simulink model "SdbTxSigProc"  (R2022b) (continued)
== system SdbTxSigProc == (continued)
  HeatrAirTReqFirstLe_HeatrAirTReqFirstLe : Outport  Port=63  OutMin=-60  OutMax=195  OutDataTypeStr=T4  PortDimensions=1
  HeatrAirTReqFirstRi_HeatrAirTReqFirstRi : Outport  Port=64  OutMin=-60  OutMax=195  OutDataTypeStr=T4  PortDimensions=1
  HeatrAirTReqSecLe_HeatrAirTReqSecLe : Outport  Port=65  OutMin=-60  OutMax=195  OutDataTypeStr=T4  PortDimensions=1
  HighTempCleanRunningSts_HighTempCleanRunningSts : Outport  Port=66  OutDataTypeStr=Enum: OnOffNoReq  PortDimensions=1
  HighTempCleanSettingSts_HighTempCleanSettingSts : Outport  Port=67  OutDataTypeStr=Enum: OnOffNoReq  PortDimensions=1
  HvacAirTForRowThrdAtFlrRi_HvacAirTForRowThrdAtFlrRi : Outport  Port=68  OutDataTypeStr=Bus: EvaprTFrnt  PortDimensions=1  BusOutputAsStruct=on
  HvacAirTForRowThrdAtVentRi_HvacAirTForRowThrdAtVentRi : Outport  Port=69  OutDataTypeStr=Bus: EvaprTFrnt  PortDimensions=1  BusOutputAsStruct=on
  HvacEvaprTSpRe_HvacEvaprTSpRe : Outport  Port=70  OutDataTypeStr=HvacEvaprTSp  PortDimensions=1
  HvacFanLvlFbFrnt_HvacFanLvlFbFrnt : Outport  Port=71  OutDataTypeStr=Enum: HmiHvacFanLvl  PortDimensions=1
  HvacFanLvlFbRe_HvacFanLvlFbRe : Outport  Port=72  OutDataTypeStr=Enum: HmiHvacFanLvl  PortDimensions=1
  HvacHeatElmAirMFlowEstimdFirstLe_HvacHeatElmAirMFlowEstimdFirstLe : Outport  Port=73  OutMin=0  OutMax=1000  OutDataTypeStr=MFlow1  PortDimensions=1
  HvacHeatElmAirMFlowEstimdFirstRi_HvacHeatElmAirMFlowEstimdFirstRi : Outport  Port=74  OutMin=0  OutMax=1000  OutDataTypeStr=MFlow1  PortDimensions=1
  HvacHeatElmAirMFlowEstimdSecLe_HvacHeatElmAirMFlowEstimdSecLe : Outport  Port=75  OutMin=0  OutMax=1000  OutDataTypeStr=MFlow1  PortDimensions=1
  HvacHexAirTFirstLe_HvacHexAirTFirstLe : Outport  Port=76  OutDataTypeStr=Bus: HvacAirTForHeatrFrnt  PortDimensions=1  BusOutputAsStruct=on
  HvacHexAirTFirstRi_HvacHexAirTFirstRi : Outport  Port=77  OutDataTypeStr=Bus: HvacAirTForHeatrFrnt  PortDimensions=1  BusOutputAsStruct=on
  HvacHexAirTSecLe_HvacHexAirTSecLe : Outport  Port=78  OutDataTypeStr=Bus: HvacAirTForHeatrFrnt  PortDimensions=1  BusOutputAsStruct=on
  HvahAirTsp_HvahAirTsp : Outport  Port=79  OutDataTypeStr=TByCmptmtSnsrRelHum  PortDimensions=1
  HvahHeatgReq_HvahHeatgReq : Outport  Port=80  OutDataTypeStr=Enum: OnOff1  PortDimensions=1
  HwFrntBlwPwmReq_HwFrntBlwPwmReq : Outport  Port=81  OutMin=0  OutMax=100  OutDataTypeStr=Perc9  PortDimensions=1
  KeySynchrnztnLcl_KeySynchrnztnLcl : Outport  Port=82  OutDataTypeStr=Bus: RemSynchrnztnLcl  PortDimensions=1  BusOutputAsStruct=on
  MistRiskLvl_MistRiskLvl : Outport  Port=83  OutDataTypeStr=Perc4  PortDimensions=1
  OutlSovActvSts_OutlSovActvSts : Outport  Port=84  OutDataTypeStr=Enum: OnOff1  PortDimensions=1
  OxygenConcSnsrActnReq_OxygenConcSnsrActnReq : Outport  Port=85  OutDataTypeStr=Enum: OnOff1  PortDimensions=1
  OxygenConcSnsrStsFrmClima_OxygenConcSnsrStsFrmClima : Outport  Port=86  OutDataTypeStr=Enum: PmSnsrSts  PortDimensions=1
  OxygenSplyAvlStsFrmClima_OxygenSplyAvlStsFrmClima : Outport  Port=87  OutDataTypeStr=Enum: OxygenSplyAvlSts  PortDimensions=1
  OxygenSplyErrFrmClima_OxygenSplyErrFrmClima : Outport  Port=88  OutDataTypeStr=Enum: Err1  PortDimensions=1
  OxygenSplyPopUpReq_OxygenSplyPopUpReq : Outport  Port=89  OutDataTypeStr=Enum: OxygenSplyPopUpReq  PortDimensions=1
  OxygenSplyPwrAllwd_OxygenSplyPwrAllwd : Outport  Port=90  OutMin=0  OutMax=2047  OutDataTypeStr=Pwr30  PortDimensions=1
  OxygenSplyReq_OxygenSplyReq : Outport  Port=91  OutDataTypeStr=Enum: OxygenSplyReq  PortDimensions=1
  OxygenSplyReqFaild_OxygenSplyReqFaild : Outport  Port=92  OutMin=0  OutMax=3  OutDataTypeStr=UInt2  PortDimensions=1
  OxygenSplyVoiceFb_OxygenSplyVoiceFb : Outport  Port=93  OutDataTypeStr=Enum: OxygenSplyVoiceFb  PortDimensions=1
  PrkgClimaEngStReq_PrkgClimaEngStReq : Outport  Port=94  OutDataTypeStr=Enum: OnOff1  PortDimensions=1
  PwrCnsForHvacFanFrnt_PwrCnsForHvacFanFrnt : Outport  Port=95  OutMin=0  OutMax=2047  OutDataTypeStr=Pwr30  PortDimensions=1
  PwrCnsForHvacFanRe_PwrCnsForHvacFanRe : Outport  Port=96  OutMin=0  OutMax=2047  OutDataTypeStr=Pwr30  PortDimensions=1
  ReClimaManModCmftSts_ReClimaManModCmftSts : Outport  Port=97  OutDataTypeStr=Enum: ClimaManModCmftSts  PortDimensions=1
  RemClimaElecDefrstReq_RemClimaElecDefrstReq : Outport  Port=98  OutDataTypeStr=Bus: DefrstReq  PortDimensions=1  BusOutputAsStruct=on
  RemOxygenSplyActvFaild_RemOxygenSplyActvFaild : Outport  Port=99  OutMin=0  OutMax=3  OutDataTypeStr=UInt2  PortDimensions=1
  TCSCmprStrtFlt_TCSCmprStrtFlt : Outport  Port=100  OutDataTypeStr=Enum: OnOff1  PortDimensions=1
  TCSCmprStrtFlt1_TCSCmprStrtFlt1 : Outport  Port=101  OutDataTypeStr=Enum: OnOff1  PortDimensions=1
  TCScoolgGrdReq_TCScoolgGrdReq : Outport  Port=102  OutMin=0  OutMax=15  OutDataTypeStr=UInt4  PortDimensions=1
  TCScoolgGrdReq1_TCScoolgGrdReq1 : Outport  Port=103  OutMin=0  OutMax=15  OutDataTypeStr=UInt4  PortDimensions=1
  TCSCooltFlowReq1_TCSCooltFlowReq1 : Outport  Port=104  OutDataTypeStr=CooltFlow1  PortDimensions=1
  TCSCooltTReq1_TCSCooltTReq1 : Outport  Port=105  OutDataTypeStr=TempAtTime  PortDimensions=1
  TCSDoorSts_TCSDoorSts : Outport  Port=106  OutDataTypeStr=Enum: OnOff1  PortDimensions=1
  TCSDoorSts1_TCSDoorSts1 : Outport  Port=107  OutDataTypeStr=Enum: OnOff1  PortDimensions=1
  TCSDoorTopSts1_TCSDoorTopSts1 : Outport  Port=108  OutDataTypeStr=Enum: WhlMotSysCluReq  PortDimensions=1
  TcsEcoModSts1_Element : Outport  Port=109  OutDataTypeStr=Enum: OnOff1  PortDimensions=1
  TcsFltNewSts1_TcsFltNewSts1 : Outport  Port=110  OutDataTypeStr=Bus: Boolean32  PortDimensions=1  BusOutputAsStruct=on
  TCSFltSts_TCSFltSts : Outport  Port=111  OutMin=0  OutMax=15  OutDataTypeStr=UInt4  PortDimensions=1
  TCSFltSts1_TCSFltSts1 : Outport  Port=112  OutMin=0  OutMax=15  OutDataTypeStr=UInt4  PortDimensions=1
  TCSModSwtSts_TCSModSwtSts : Outport  Port=113  OutDataTypeStr=Enum: TelmThermoTankReq  PortDimensions=1
  TCSModSwtSts1_TCSModSwtSts1 : Outport  Port=114  OutDataTypeStr=Enum: TelmThermoTankReq  PortDimensions=1
  TCSnsrTSts_TCSnsrTSts : Outport  Port=115  OutDataTypeStr=TempAtTime  PortDimensions=1
  TCSnsrTSts1_TCSnsrTSts1 : Outport  Port=116  OutDataTypeStr=TempAtTime  PortDimensions=1
  TCSRadrTreq_TCSRadrTreq : Outport  Port=117  OutDataTypeStr=TempAtTime  PortDimensions=1
  TCSRadrTreq1_TCSRadrTreq1 : Outport  Port=118  OutDataTypeStr=TempAtTime  PortDimensions=1
  TCSRadrTSts_TCSRadrTSts : Outport  Port=119  OutDataTypeStr=TempAtTime  PortDimensions=1
  TCSRadrTSts1_TCSRadrTSts1 : Outport  Port=120  OutDataTypeStr=TempAtTime  PortDimensions=1
  TCSTmrOverSts_TCSTmrOverSts : Outport  Port=121  OutMin=0  OutMax=31  OutDataTypeStr=TimeHr5  PortDimensions=1
  TCSTmrOverSts1_TCSTmrOverSts1 : Outport  Port=122  OutMin=0  OutMax=31  OutDataTypeStr=TimeHr5  PortDimensions=1
  TCSTmrStrtSts_TCSTmrStrtSts : Outport  Port=123  OutDataTypeStr=Enum: OnOffNoReq  PortDimensions=1
  TCSTmrStrtSts1_TCSTmrStrtSts1 : Outport  Port=124  OutDataTypeStr=Enum: OnOffNoReq  PortDimensions=1
  TCSTNowSts_TCSTNowSts : Outport  Port=125  OutDataTypeStr=TempAtTime  PortDimensions=1
  TCSTNowSts1_TCSTNowSts1 : Outport  Port=126  OutDataTypeStr=TempAtTime  PortDimensions=1
  TgtHvacAirTForMaxTZone_TgtHvacAirTForMaxTZone : Outport  Port=127  OutMin=-256  OutMax=255.9  OutDataTypeStr=T2  PortDimensions=1
  ThermoTSteDeoSts1_ThermoTSteDeoSts1 : Outport  Port=128  OutDataTypeStr=UInt8  PortDimensions=1
  ThrdRowLeSeatHeatgPwrAllwd_ThrdRowLeSeatHeatgPwrAllwd : Outport  Port=129  OutDataTypeStr=UInt8  PortDimensions=1
  ThrdRowLeSeatVentnPwrAllwd_ThrdRowLeSeatVentnPwrAllwd : Outport  Port=130  OutDataTypeStr=UInt8  PortDimensions=1
  ThrdRowRiSeatHeatgPwrAllwd_ThrdRowRiSeatHeatgPwrAllwd : Outport  Port=131  OutDataTypeStr=UInt8  PortDimensions=1
  ThrdRowRiSeatVentnPwrAllwd_ThrdRowRiSeatVentnPwrAllwd : Outport  Port=132  OutDataTypeStr=UInt8  PortDimensions=1
  TiOfDeactvnForClimaCtrl_TiOfDeactvnForClimaCtrl : Outport  Port=133  OutMin=0  OutMax=59  OutDataTypeStr=Sec1  PortDimensions=1
  VMMChngNdMtn_VMMChngNdMtn : Outport  Port=134  OutDataTypeStr=Enum: OnOff1  PortDimensions=1
  AirTFbFromClima_AirTFbFromClima : Outport  Port=135  OutDataTypeStr=Bus: AirTFbFromClimaRec  PortDimensions=1  BusOutputAsStruct=on
  CarTiIntForClima_CarTiIntForClima : Outport  Port=136  OutDataTypeStr=CarTiGlb  PortDimensions=1
  ClimaActv_ClimaActv : Outport  Port=137  OutDataTypeStr=Enum: OnOff1  PortDimensions=1
  ClimaActvAlrmSts_ClimaActvAlrmSts : Outport  Port=138  OutDataTypeStr=Enum: OnOff1  PortDimensions=1
  ClimaCmptSts_ClimaCmptSts : Outport  Port=139  OutDataTypeStr=Enum: CmptSts  PortDimensions=1
  ClimaDefrstSts_ClimaDefrstSts : Outport  Port=140  OutDataTypeStr=Enum: OnOff1  PortDimensions=1
  ClimaOffReq_ClimaOffReq : Outport  Port=141  OutDataTypeStr=Enum: ClimaOffReq  PortDimensions=1
  ClimaPwrCns_ClimaPwrCns : Outport  Port=142  OutMin=0  OutMax=2047  OutDataTypeStr=Pwr30  PortDimensions=1
  ClimaPwrCritDes_ClimaPwrCritDes : Outport  Port=143  OutMin=0  OutMax=2047  OutDataTypeStr=Pwr30  PortDimensions=1
  ClimaPwrNormDes_ClimaPwrNormDes : Outport  Port=144  OutMin=0  OutMax=2047  OutDataTypeStr=Pwr30  PortDimensions=1
  ClimaTiOfEnd_ClimaTiOfEnd : Outport  Port=145  OutDataTypeStr=CarTiGlb  PortDimensions=1
  CmptModForHp_CmptModForHp : Outport  Port=146  OutDataTypeStr=Enum: ModeCmptHp  PortDimensions=1
  CupHoldrActvAllwd_CupHoldrActvAllwd : Outport  Port=147  OutDataTypeStr=ScrHeatrEgyPwrCns1  PortDimensions=1
  DefrstMaxSts_DefrstMaxSts : Outport  Port=148  OutDataTypeStr=Boolean  PortDimensions=1
  DrvrSeatHeatgPwrAllwd_DrvrSeatHeatgPwrAllwd : Outport  Port=149  OutDataTypeStr=UInt8  PortDimensions=1
  DrvrSeatVentnPwrAllwd_DrvrSeatVentnPwrAllwd : Outport  Port=150  OutDataTypeStr=UInt8  PortDimensions=1
  EcoClimaSts_EcoClimaSts : Outport  Port=151  OutDataTypeStr=Enum: OnOff1  PortDimensions=1
  EgyDesForClima_EgyDesForClima : Outport  Port=152  OutDataTypeStr=Egy2  PortDimensions=1
  ElecDefrstReq_ElecDefrstReq : Outport  Port=153  OutDataTypeStr=Bus: DefrstReq  PortDimensions=1  BusOutputAsStruct=on
  EngRunngReqByClima_EngRunngReqByClima : Outport  Port=154  OutDataTypeStr=Enum: EngRunngReq  PortDimensions=1
  EngSpdIncIdleForClima_EngSpdIncIdleForClima : Outport  Port=155  OutDataTypeStr=Enum: Flg1  PortDimensions=1
  EvaprTFrnt_EvaprTFrnt : Outport  Port=156  OutDataTypeStr=Bus: EvaprTFrnt  PortDimensions=1  BusOutputAsStruct=on
  ExtVentToastFrmClima_ExtVentToastFrmClima : Outport  Port=157  OutDataTypeStr=Enum: ExtVentToastFrmClimaSts  PortDimensions=1
  HvacAirMFlowEstimd_HvacAirMFlowEstimd : Outport  Port=158  OutMin=0  OutMax=1000  OutDataTypeStr=MFlow1  PortDimensions=1
  HvacAirMFlowReEstimd_HvacAirMFlowReEstimd : Outport  Port=159  OutMin=0  OutMax=1000  OutDataTypeStr=MFlow1  PortDimensions=1
  HvacAirMFlowVent_HvacAirMFlowVent : Outport  Port=160  OutMin=0  OutMax=1000  OutDataTypeStr=MFlow1  PortDimensions=1
  HvacAirTForRowFirstAtFlrLe_HvacAirTForRowFirstAtFlrLe : Outport  Port=161  OutDataTypeStr=Bus: EvaprTFrnt  PortDimensions=1  BusOutputAsStruct=on
  HvacAirTForRowFirstAtFlrRi_HvacAirTForRowFirstAtFlrRi : Outport  Port=162  OutDataTypeStr=Bus: EvaprTFrnt  PortDimensions=1  BusOutputAsStruct=on
  HvacAirTForRowFirstAtVentLe_HvacAirTForRowFirstAtVentLe : Outport  Port=163  OutDataTypeStr=Bus: EvaprTFrnt  PortDimensions=1  BusOutputAsStruct=on
  HvacAirTForRowFirstAtVentRi_HvacAirTForRowFirstAtVentRi : Outport  Port=164  OutDataTypeStr=Bus: EvaprTFrnt  PortDimensions=1  BusOutputAsStruct=on
  HvacAirTForRowSecAtFlrLe_HvacAirTForRowSecAtFlrLe : Outport  Port=165  OutDataTypeStr=Bus: EvaprTFrnt  PortDimensions=1  BusOutputAsStruct=on
  HvacAirTForRowSecAtFlrRi_HvacAirTForRowSecAtFlrRi : Outport  Port=166  OutDataTypeStr=Bus: EvaprTFrnt  PortDimensions=1  BusOutputAsStruct=on
  HvacAirTForRowSecAtVentLe_HvacAirTForRowSecAtVentLe : Outport  Port=167  OutDataTypeStr=Bus: EvaprTFrnt  PortDimensions=1  BusOutputAsStruct=on
  HvacAirTForRowSecAtVentRi_HvacAirTForRowSecAtVentRi : Outport  Port=168  OutDataTypeStr=Bus: EvaprTFrnt  PortDimensions=1  BusOutputAsStruct=on
  HvacCondsorTsp_HvacCondsorTsp : Outport  Port=169  OutDataTypeStr=Bus: EvaprTFrnt  PortDimensions=1  BusOutputAsStruct=on
  HvacCoolgEnaFrnt_HvacCoolgEnaFrnt : Outport  Port=170  OutDataTypeStr=Enum: OnOff1  PortDimensions=1
  HvacCoolgEnaRe_HvacCoolgEnaRe : Outport  Port=171  OutDataTypeStr=Enum: OnOff1  PortDimensions=1
  HvacHeatElmAirMFlowEstimd_HvacHeatElmAirMFlowEstimd : Outport  Port=172  OutMin=0  OutMax=1000  OutDataTypeStr=MFlow1  PortDimensions=1
  HvacHeatgReq_HvacHeatgReq : Outport  Port=173  OutDataTypeStr=Enum: OnOff1  PortDimensions=1
  HvacRecircAct_HvacRecircAct : Outport  Port=174  OutDataTypeStr=Perc4  PortDimensions=1
  HvEgyDesForClima_HvEgyDesForClima : Outport  Port=175  OutDataTypeStr=HvEgyDesForClima  PortDimensions=1
  InPm25SnsrActnReq_InPm25SnsrActnReq : Outport  Port=176  OutDataTypeStr=Enum: OnOff1  PortDimensions=1
  IntPm25HiPopUp_IntPm25HiPopUp : Outport  Port=177  OutDataTypeStr=Enum: IntPmHiPopUp  PortDimensions=1
  LvlOfClimaCmft_LvlOfClimaCmft : Outport  Port=178  OutDataTypeStr=Enum: LvlOfClimaCmft  PortDimensions=1
  PassSeatHeatgPwrAllwd_PassSeatHeatgPwrAllwd : Outport  Port=179  OutDataTypeStr=UInt8  PortDimensions=1
  PassSeatVentnPwrAllwd_PassSeatVentnPwrAllwd : Outport  Port=180  OutDataTypeStr=UInt8  PortDimensions=1
  PostDrvgClimaAvl_PostDrvgClimaAvl : Outport  Port=181  OutDataTypeStr=Enum: OnOff1  PortDimensions=1
  PwrAllwdForElecAirVentn_PwrAllwdForElecAirVentn : Outport  Port=182  OutDataTypeStr=ScrHeatrEgyPwrCns1  PortDimensions=1
  PwrEstimdAtStbForClima_PwrEstimdAtStbForClima : Outport  Port=183  OutMin=0  OutMax=2047  OutDataTypeStr=Pwr30  PortDimensions=1
  RlyCmftForClimaReq_RlyCmftForClimaReq : Outport  Port=184  OutDataTypeStr=Enum: OnOff1  PortDimensions=1
  RlyCrashForClimaReq_RlyCrashForClimaReq : Outport  Port=185  OutDataTypeStr=Enum: OnOff1  PortDimensions=1
  SecRowLeSeatHeatgPwrAllwd_SecRowLeSeatHeatgPwrAllwd : Outport  Port=186  OutDataTypeStr=UInt8  PortDimensions=1
  SecRowLeSeatVentnPwrAllwd_SecRowLeSeatVentnPwrAllwd : Outport  Port=187  OutDataTypeStr=UInt8  PortDimensions=1
  SecRowRiSeatHeatgPwrAllwd_SecRowRiSeatHeatgPwrAllwd : Outport  Port=188  OutDataTypeStr=UInt8  PortDimensions=1
  SecRowRiSeatVentnPwrAllwd_SecRowRiSeatVentnPwrAllwd : Outport  Port=189  OutDataTypeStr=UInt8  PortDimensions=1
  SteerWhlHeatgDurgClima_SteerWhlHeatgDurgClima : Outport  Port=190  OutDataTypeStr=Boolean  PortDimensions=1
  TgtHvacAirTForRowFirstLe_TgtHvacAirTForRowFirstLe : Outport  Port=191  OutMin=-256  OutMax=255.9  OutDataTypeStr=T2  PortDimensions=1
  TgtHvacAirTForRowFirstRi_TgtHvacAirTForRowFirstRi : Outport  Port=192  OutMin=-256  OutMax=255.9  OutDataTypeStr=T2  PortDimensions=1
  TgtHvacAirTForRowSecLe_TgtHvacAirTForRowSecLe : Outport  Port=193  OutMin=-256  OutMax=255.9  OutDataTypeStr=T2  PortDimensions=1
  TgtHvacAirTForRowSecRi_TgtHvacAirTForRowSecRi : Outport  Port=194  OutMin=-256  OutMax=255.9  OutDataTypeStr=T2  PortDimensions=1
  UBoostReqByClima_UBoostReqByClima : Outport  Port=195  OutDataTypeStr=Enum: ReqSts1  PortDimensions=1
  VehMktForPmSnsr_VehMktForPmSnsr : Outport  Port=196  OutDataTypeStr=Bus: AirQlyMeasInValReq  PortDimensions=1  BusOutputAsStruct=on
  WindIceDetdFrnt_WindIceDetdFrnt : Outport  Port=197  OutDataTypeStr=Enum: OnOff1  PortDimensions=1
  WindIceDetdRe_WindIceDetdRe : Outport  Port=198  OutDataTypeStr=Enum: OnOff1  PortDimensions=1
  wires:
  AirTAftHvacDes_AirTAftHvacDes/1 -> SdbTxSigProc_Runnable_sys/1(AirTAftHvacDes_AirTAftHvacDes_read)
  AirTFbFromClima_Ngcc_AirTFbFromClima/1 -> SdbTxSigProc_Runnable_sys/2(AirTFbFromClima_Ngcc_AirTFbFromClima_read)
  AmbTEstimd_Ngcc_AmbTEstimd/1 -> SdbTxSigProc_Runnable_sys/3(AmbTEstimd_Ngcc_AmbTEstimd_read)
  AmbTFildForClimaNgcc_AmbTFildForClima/1 -> SdbTxSigProc_Runnable_sys/4(AmbTFildForClimaNgcc_AmbTFildForClima_read)
  AmbTFildForClimaQf_AmbTFildForClimaQf/1 -> SdbTxSigProc_Runnable_sys/5(AmbTFildForClimaQf_AmbTFildForClimaQf_read)
  CdsnProbltyToExt_CdsnProbltyToExt/1 -> SdbTxSigProc_Runnable_sys/6(CdsnProbltyToExt_CdsnProbltyToExt_read)
  ClimaActv_Ngcc_ClimaActv/1 -> SdbTxSigProc_Runnable_sys/7(ClimaActv_Ngcc_ClimaActv_read)
  ClimaActvAlrmSts_Ngcc_ClimaActvAlrmSts/1 -> SdbTxSigProc_Runnable_sys/8(ClimaActvAlrmSts_Ngcc_ClimaActvAlrmSts_read)
  ClimaCoolgPwrThermPred_ClimaCoolgPwrThermPred/1 -> SdbTxSigProc_Runnable_sys/9(ClimaCoolgPwrThermPred_ClimaCoolgPwrThermPred_read)
  ClimaDefrstMaxReq_ClimaDefrstMaxReq/1 -> SdbTxSigProc_Runnable_sys/10(ClimaDefrstMaxReq_ClimaDefrstMaxReq_read)
  ClimaOffReq_Ngcc_ClimaOffReq/1 -> SdbTxSigProc_Runnable_sys/11(ClimaOffReq_Ngcc_ClimaOffReq_read)
  ClimaPwrCns_Ngcc_ClimaPwrCns/1 -> SdbTxSigProc_Runnable_sys/12(ClimaPwrCns_Ngcc_ClimaPwrCns_read)
  ClimaPwrCnsPred_ClimaPwrCnsPred/1 -> SdbTxSigProc_Runnable_sys/13(ClimaPwrCnsPred_ClimaPwrCnsPred_read)
  ClimaPwrCritDes_Ngcc_ClimaPwrCritDes/1 -> SdbTxSigProc_Runnable_sys/14(ClimaPwrCritDes_Ngcc_ClimaPwrCritDes_read)
  ClimaPwrNormDes_Ngcc_ClimaPwrNormDes/1 -> SdbTxSigProc_Runnable_sys/15(ClimaPwrNormDes_Ngcc_ClimaPwrNormDes_read)
  ClimaStrtTi_ClimaStrtTi/1 -> SdbTxSigProc_Runnable_sys/16(ClimaStrtTi_ClimaStrtTi_read)
  ClimaSts_Ngcc_ClimaSts/1 -> SdbTxSigProc_Runnable_sys/17(ClimaSts_Ngcc_ClimaSts_read)
  ClimaTiOfEnd_Ngcc_ClimaTiOfEnd/1 -> SdbTxSigProc_Runnable_sys/18(ClimaTiOfEnd_Ngcc_ClimaTiOfEnd_read)
  ClimaTmrSts_ClimaTmrSts/1 -> SdbTxSigProc_Runnable_sys/19(ClimaTmrSts_ClimaTmrSts_read)
  ClimaWarn_ClimaWarn/1 -> SdbTxSigProc_Runnable_sys/20(ClimaWarn_ClimaWarn_read)
  ClngActv_ClngActv/1 -> SdbTxSigProc_Runnable_sys/21(ClngActv_ClngActv_read)
  CmptmtAirTEstimdAtRowFirstLoResl_Ngcc_CmptmtAirTEstimdAtRowFirstLoResl/1 -> SdbTxSigProc_Runnable_sys/22(CmptmtAirTEstimdAtRowFirstLoResl_Ngcc_CmptmtAirTEstimdAtRowFirstLoResl_read)
  CmptmtAirTEstimdExtd_Ngcc_CmptmtAirTEstimdExtd/1 -> SdbTxSigProc_Runnable_sys/23(CmptmtAirTEstimdExtd_Ngcc_CmptmtAirTEstimdExtd_read)
  CmptmtAirTFbExtd_CmptmtAirTFbExtd/1 -> SdbTxSigProc_Runnable_sys/24(CmptmtAirTFbExtd_CmptmtAirTFbExtd_read)
  CmptmtRelHumToExt_CmptmtRelHumToExt/1 -> SdbTxSigProc_Runnable_sys/25(CmptmtRelHumToExt_CmptmtRelHumToExt_read)
  CoolgStsForDisp_CoolgStsForDisp/1 -> SdbTxSigProc_Runnable_sys/26(CoolgStsForDisp_CoolgStsForDisp_read)
  CupHoldrActvAllwd_Ngcc_CupHoldrActvAllwd/1 -> SdbTxSigProc_Runnable_sys/27(CupHoldrActvAllwd_Ngcc_CupHoldrActvAllwd_read)
  CustClimaOvrdNotifForHmiCpt_CustClimaOvrdNotifForHmiCpt/1 -> SdbTxSigProc_Runnable_sys/28(CustClimaOvrdNotifForHmiCpt_CustClimaOvrdNotifForHmiCpt_read)
  DefrstReq_DefrstReq/1 -> SdbTxSigProc_Runnable_sys/29(DefrstReq_DefrstReq_read)
  DewVal_DewVal/1 -> SdbTxSigProc_Runnable_sys/30(DewVal_DewVal_read)
  DrvrSeatHeatgPwrAllwd_Ngcc_DrvrSeatHeatgPwrAllwd/1 -> SdbTxSigProc_Runnable_sys/31(DrvrSeatHeatgPwrAllwd_Ngcc_DrvrSeatHeatgPwrAllwd_read)
  DrvrSeatVentnPwrAllwd_Ngcc_DrvrSeatVentnPwrAllwd/1 -> SdbTxSigProc_Runnable_sys/32(DrvrSeatVentnPwrAllwd_Ngcc_DrvrSeatVentnPwrAllwd_read)
  EcoClimasts_Ngcc_EcoClimasts/1 -> SdbTxSigProc_Runnable_sys/33(EcoClimasts_Ngcc_EcoClimasts_read)
  EcoModForClimaActv_EcoModForClimaActv/1 -> SdbTxSigProc_Runnable_sys/34(EcoModForClimaActv_EcoModForClimaActv_read)
  EgyDesForClima_Ngcc_EgyDesForClima/1 -> SdbTxSigProc_Runnable_sys/35(EgyDesForClima_Ngcc_EgyDesForClima_read)
  EngRunngReqByClima_Ngcc_EngRunngReqByClima/1 -> SdbTxSigProc_Runnable_sys/36(EngRunngReqByClima_Ngcc_EngRunngReqByClima_read)
  EngSpdIncIdleForClima_Ngcc_EngSpdIncIdleForClima/1 -> SdbTxSigProc_Runnable_sys/37(EngSpdIncIdleForClima_Ngcc_EngSpdIncIdleForClima_read)
  EvaprTFrntFildForClima_EvaprTFrntFildForClima/1 -> SdbTxSigProc_Runnable_sys/38(EvaprTFrntFildForClima_EvaprTFrntFildForClima_read)
  EvaprTFrntFildQfForClima_EvaprTFrntFildQfForClima/1 -> SdbTxSigProc_Runnable_sys/39(EvaprTFrntFildQfForClima_EvaprTFrntFildQfForClima_read)
  HmiDefrstReqForClima_HmiDefrstReqForClima/1 -> SdbTxSigProc_Runnable_sys/40(HmiDefrstReqForClima_HmiDefrstReqForClima_read)
  HvacAirMFlowEstimd_Ngcc_HvacAirMFlowEstimd/1 -> SdbTxSigProc_Runnable_sys/41(HvacAirMFlowEstimd_Ngcc_HvacAirMFlowEstimd_read)
  HvacAirMFlowEstimdInHeatElm_HvacAirMFlowEstimdInHeatElm/1 -> SdbTxSigProc_Runnable_sys/42(HvacAirMFlowEstimdInHeatElm_HvacAirMFlowEstimdInHeatElm_read)
  HvacAirMFlowReEstimd_Ngcc_HvacAirMFlowReEstimd/1 -> SdbTxSigProc_Runnable_sys/43(HvacAirMFlowReEstimd_Ngcc_HvacAirMFlowReEstimd_read)
  HvacAirTFildForRowFirstAtFlrLe_HvacAirTFildForRowFirstAtFlrLe/1 -> SdbTxSigProc_Runnable_sys/44(HvacAirTFildForRowFirstAtFlrLe_HvacAirTFildForRowFirstAtFlrLe_read)
  HvacAirTFildForRowFirstAtFlrRi_HvacAirTFildForRowFirstAtFlrRi/1 -> SdbTxSigProc_Runnable_sys/45(HvacAirTFildForRowFirstAtFlrRi_HvacAirTFildForRowFirstAtFlrRi_read)
  HvacAirTFildForRowFirstAtVentLe_HvacAirTFildForRowFirstAtVentLe/1 -> SdbTxSigProc_Runnable_sys/46(HvacAirTFildForRowFirstAtVentLe_HvacAirTFildForRowFirstAtVentLe_read)
  HvacAirTFildForRowFirstAtVentRi_HvacAirTFildForRowFirstAtVentRi/1 -> SdbTxSigProc_Runnable_sys/47(HvacAirTFildForRowFirstAtVentRi_HvacAirTFildForRowFirstAtVentRi_read)
  HvacAirTFildForRowSecAtFlrLe_HvacAirTFildForRowSecAtFlrLe/1 -> SdbTxSigProc_Runnable_sys/48(HvacAirTFildForRowSecAtFlrLe_HvacAirTFildForRowSecAtFlrLe_read)
  HvacAirTFildForRowSecAtFlrRi_HvacAirTFildForRowSecAtFlrRi/1 -> SdbTxSigProc_Runnable_sys/49(HvacAirTFildForRowSecAtFlrRi_HvacAirTFildForRowSecAtFlrRi_read)
  HvacAirTFildForRowSecAtVentLe_HvacAirTFildForRowSecAtVentLe/1 -> SdbTxSigProc_Runnable_sys/50(HvacAirTFildForRowSecAtVentLe_HvacAirTFildForRowSecAtVentLe_read)
  HvacAirTFildForRowSecAtVentRi_HvacAirTFildForRowSecAtVentRi/1 -> SdbTxSigProc_Runnable_sys/51(HvacAirTFildForRowSecAtVentRi_HvacAirTFildForRowSecAtVentRi_read)
  HvacAirTForRowFirstAtFlrLeRaw_HvacAirTForRowFirstAtFlrLeRaw/1 -> SdbTxSigProc_Runnable_sys/52(HvacAirTForRowFirstAtFlrLeRaw_HvacAirTForRowFirstAtFlrLeRaw_read)
  HvacAirTForRowFirstAtFlrRiRaw_HvacAirTForRowFirstAtFlrRiRaw/1 -> SdbTxSigProc_Runnable_sys/53(HvacAirTForRowFirstAtFlrRiRaw_HvacAirTForRowFirstAtFlrRiRaw_read)
  HvacAirTForRowFirstAtVentRiRaw_HvacAirTForRowFirstAtVentRiRaw/1 -> SdbTxSigProc_Runnable_sys/54(HvacAirTForRowFirstAtVentRiRaw_HvacAirTForRowFirstAtVentRiRaw_read)
  HvacAirTForRowSecAtFlrLeRaw_HvacAirTForRowSecAtFlrLeRaw/1 -> SdbTxSigProc_Runnable_sys/55(HvacAirTForRowSecAtFlrLeRaw_HvacAirTForRowSecAtFlrLeRaw_read)
  HvacAirTForRowSecAtFlrRiRaw_HvacAirTForRowSecAtFlrRiRaw/1 -> SdbTxSigProc_Runnable_sys/56(HvacAirTForRowSecAtFlrRiRaw_HvacAirTForRowSecAtFlrRiRaw_read)
  HvacAirTForRowSecAtVentLeRaw_HvacAirTForRowSecAtVentLeRaw/1 -> SdbTxSigProc_Runnable_sys/57(HvacAirTForRowSecAtVentLeRaw_HvacAirTForRowSecAtVentLeRaw_read)
  HvacAirTForRowSecAtVentRiRaw_HvacAirTForRowSecAtVentRiRaw/1 -> SdbTxSigProc_Runnable_sys/58(HvacAirTForRowSecAtVentRiRaw_HvacAirTForRowSecAtVentRiRaw_read)
  HvacAirTReqAtRowFirstLe_HvacAirTReqAtRowFirstLe/1 -> SdbTxSigProc_Runnable_sys/59(HvacAirTReqAtRowFirstLe_HvacAirTReqAtRowFirstLe_read)
  HvacAirTReqAtRowFirstRi_HvacAirTReqAtRowFirstRi/1 -> SdbTxSigProc_Runnable_sys/60(HvacAirTReqAtRowFirstRi_HvacAirTReqAtRowFirstRi_read)
  HvacAirTReqAtRowSecLe_HvacAirTReqAtRowSecLe/1 -> SdbTxSigProc_Runnable_sys/61(HvacAirTReqAtRowSecLe_HvacAirTReqAtRowSecLe_read)
  HvacAirTReqAtRowSecRi_HvacAirTReqAtRowSecRi/1 -> SdbTxSigProc_Runnable_sys/62(HvacAirTReqAtRowSecRi_HvacAirTReqAtRowSecRi_read)
  HvacCondsorTspEvaprTFrnt_HvacCondsorTspEvaprTFrnt/1 -> SdbTxSigProc_Runnable_sys/63(HvacCondsorTspEvaprTFrnt_HvacCondsorTspEvaprTFrnt_read)
  HvacCoolgEnaFrnt_Ngcc_HvacCoolgEnaFrnt/1 -> SdbTxSigProc_Runnable_sys/64(HvacCoolgEnaFrnt_Ngcc_HvacCoolgEnaFrnt_read)
  HvacCoolgEnaRe_Ngcc_HvacCoolgEnaRe/1 -> SdbTxSigProc_Runnable_sys/65(HvacCoolgEnaRe_Ngcc_HvacCoolgEnaRe_read)
  HvacEvaprTSpFrnt_Ngcc_HvacEvaprTSpFrnt/1 -> SdbTxSigProc_Runnable_sys/66(HvacEvaprTSpFrnt_Ngcc_HvacEvaprTSpFrnt_read)
  HvacFlowFrntActForRowFirstAtVent_HvacFlowFrntActForRowFirstAtVent/1 -> SdbTxSigProc_Runnable_sys/67(HvacFlowFrntActForRowFirstAtVent_HvacFlowFrntActForRowFirstAtVent_read)
  HvacFlowFrntActForRowFirstAtVent_HvacFlowFrntActForRowFirstAtVentLe/1 -> SdbTxSigProc_Runnable_sys/68(HvacFlowFrntActForRowFirstAtVent_HvacFlowFrntActForRowFirstAtVentLe_read)
  HvacFlowFrntActForRowFirstAtVent_HvacFlowFrntActForRowFirstAtVentRi/1 -> SdbTxSigProc_Runnable_sys/69(HvacFlowFrntActForRowFirstAtVent_HvacFlowFrntActForRowFirstAtVentRi_read)
  HvacFlowFrntActForRowFirstAtVent_HvacFlowFrntActForRowFirstAtVentSideLe/1 -> SdbTxSigProc_Runnable_sys/70(HvacFlowFrntActForRowFirstAtVent_HvacFlowFrntActForRowFirstAtVentSideLe_read)
  HvacFlowFrntActForRowFirstAtVent_HvacFlowFrntActForRowFirstAtVentSideRi/1 -> SdbTxSigProc_Runnable_sys/71(HvacFlowFrntActForRowFirstAtVent_HvacFlowFrntActForRowFirstAtVentSideRi_read)
  HvacHeatgReqInt_HvacHeatgReqInt/1 -> SdbTxSigProc_Runnable_sys/72(HvacHeatgReqInt_HvacHeatgReqInt_read)
  HvacRecircAct_Ngcc_HvacRecircAct/1 -> SdbTxSigProc_Runnable_sys/73(HvacRecircAct_Ngcc_HvacRecircAct_read)
  HvEgyDesForClima_Ngcc_HvEgyDesForClima/1 -> SdbTxSigProc_Runnable_sys/74(HvEgyDesForClima_Ngcc_HvEgyDesForClima_read)
  InPm25SnsrActnReq_Ngcc_InPm25SnsrActnReq/1 -> SdbTxSigProc_Runnable_sys/75(InPm25SnsrActnReq_Ngcc_InPm25SnsrActnReq_read)
  IntPm25HiPopUp_Ngcc_IntPm25HiPopUp/1 -> SdbTxSigProc_Runnable_sys/76(IntPm25HiPopUp_Ngcc_IntPm25HiPopUp_read)
  LvlOfClimaCmft_Ngcc_LvlOfClimaCmft/1 -> SdbTxSigProc_Runnable_sys/77(LvlOfClimaCmft_Ngcc_LvlOfClimaCmft_read)
  PassSeatHeatgPwrAllwd_Ngcc_PassSeatHeatgPwrAllwd/1 -> SdbTxSigProc_Runnable_sys/78(PassSeatHeatgPwrAllwd_Ngcc_PassSeatHeatgPwrAllwd_read)
  PassSeatVentnPwrAllwd_Ngcc_PassSeatVentnPwrAllwd/1 -> SdbTxSigProc_Runnable_sys/79(PassSeatVentnPwrAllwd_Ngcc_PassSeatVentnPwrAllwd_read)
  PostDrvgClimaAvl_Ngcc_PostDrvgClimaAvl/1 -> SdbTxSigProc_Runnable_sys/80(PostDrvgClimaAvl_Ngcc_PostDrvgClimaAvl_read)
  PreClngActv_PreClngActv/1 -> SdbTxSigProc_Runnable_sys/81(PreClngActv_PreClngActv_read)
  PreClngNotif_PreClngNotif/1 -> SdbTxSigProc_Runnable_sys/82(PreClngNotif_PreClngNotif_read)
  PwrAllwdForElecAirVentn_Ngcc_PwrAllwdForElecAirVentn/1 -> SdbTxSigProc_Runnable_sys/83(PwrAllwdForElecAirVentn_Ngcc_PwrAllwdForElecAirVentn_read)
  PwrAllwdForSeatHeatg_PwrAllwdForSeatHeatg/1 -> SdbTxSigProc_Runnable_sys/84(PwrAllwdForSeatHeatg_PwrAllwdForSeatHeatg_read)
  PwrAllwdForSeatVent_PwrAllwdForSeatVent/1 -> SdbTxSigProc_Runnable_sys/85(PwrAllwdForSeatVent_PwrAllwdForSeatVent_read)
  PwrChrgDetdForPrkgHeatrElec_PwrChrgDetdForPrkgHeatrElec/1 -> SdbTxSigProc_Runnable_sys/86(PwrChrgDetdForPrkgHeatrElec_PwrChrgDetdForPrkgHeatrElec_read)
  PwrCnsHeatgElecPred_PwrCnsHeatgElecPred/1 -> SdbTxSigProc_Runnable_sys/87(PwrCnsHeatgElecPred_PwrCnsHeatgElecPred_read)
  PwrEstimdAtStbForClima_Ngcc_PwrEstimdAtStbForClima/1 -> SdbTxSigProc_Runnable_sys/88(PwrEstimdAtStbForClima_Ngcc_PwrEstimdAtStbForClima_read)
  RlyCmftForClimaReq_Ngcc_RlyCmftForClimaReq/1 -> SdbTxSigProc_Runnable_sys/89(RlyCmftForClimaReq_Ngcc_RlyCmftForClimaReq_read)
  RlyCrashForClimaReq_Ngcc_RlyCrashForClimaReq/1 -> SdbTxSigProc_Runnable_sys/90(RlyCrashForClimaReq_Ngcc_RlyCrashForClimaReq_read)
  SeatHeatgAutCdn_SeatHeatgAutCdn/1 -> SdbTxSigProc_Runnable_sys/91(SeatHeatgAutCdn_SeatHeatgAutCdn_read)
  SecRowLeSeatHeatgPwrAllwd_Ngcc_SecRowLeSeatHeatgPwrAllwd/1 -> SdbTxSigProc_Runnable_sys/92(SecRowLeSeatHeatgPwrAllwd_Ngcc_SecRowLeSeatHeatgPwrAllwd_read)
  SecRowLeSeatVentnPwrAllwd_Ngcc_SecRowLeSeatVentnPwrAllwd/1 -> SdbTxSigProc_Runnable_sys/93(SecRowLeSeatVentnPwrAllwd_Ngcc_SecRowLeSeatVentnPwrAllwd_read)
  SecRowRiSeatHeatgPwrAllwd_Ngcc_SecRowRiSeatHeatgPwrAllwd/1 -> SdbTxSigProc_Runnable_sys/94(SecRowRiSeatHeatgPwrAllwd_Ngcc_SecRowRiSeatHeatgPwrAllwd_read)
  SecRowRiSeatVentnPwrAllwd_Ngcc_SecRowRiSeatVentnPwrAllwd/1 -> SdbTxSigProc_Runnable_sys/95(SecRowRiSeatVentnPwrAllwd_Ngcc_SecRowRiSeatVentnPwrAllwd_read)
  SteerWhlHeatgAllwd_SteerWhlHeatgAllwd/1 -> SdbTxSigProc_Runnable_sys/96(SteerWhlHeatgAllwd_SteerWhlHeatgAllwd_read)
  SteerWhlHeatgDurgClima_Ngcc_SteerWhlHeatgDurgClima/1 -> SdbTxSigProc_Runnable_sys/97(SteerWhlHeatgDurgClima_Ngcc_SteerWhlHeatgDurgClima_read)
  UBoostReqByClima_Ngcc_UBoostReqByClima/1 -> SdbTxSigProc_Runnable_sys/98(UBoostReqByClima_Ngcc_UBoostReqByClima_read)
  VehMktForPmSnsr_Ngcc_VehMktForPmSnsr/1 -> SdbTxSigProc_Runnable_sys/99(VehMktForPmSnsr_Ngcc_VehMktForPmSnsr_read)
  WindDewUppr_WindDewTUppr/1 -> SdbTxSigProc_Runnable_sys/100(WindDewUppr_WindDewTUppr_read)
  WindIceDetdFrnt_Ngcc_WindIceDetdFrnt/1 -> SdbTxSigProc_Runnable_sys/101(WindIceDetdFrnt_Ngcc_WindIceDetdFrnt_read)
  WindIceDetdRe_Ngcc_WindIceDetdRe/1 -> SdbTxSigProc_Runnable_sys/102(WindIceDetdRe_Ngcc_WindIceDetdRe_read)
  WindT_WindT/1 -> SdbTxSigProc_Runnable_sys/103(WindT_WindT_read)
  HvacAirTForRowFirstAtVentLeRaw_HvacAirTForRowFirstAtVentLeRaw/1 -> SdbTxSigProc_Runnable_sys/104(HvacAirTForRowFirstAtVentLeRaw_HvacAirTForRowFirstAtVentLeRaw_read)
  ClimaStStrtUp_ClimaStStrtUp/1 -> SdbTxSigProc_Runnable_sys/105(ClimaStStrtUp_ClimaStStrtUp_read)
  AACAirClngActnNgcc_AACAirClngActnNgcc/1 -> SdbTxSigProc_Runnable_sys/106(AACAirClngActnNgcc_AACAirClngActnNgcc_read)
  AirSrcReqNgcc_AirSrcReqNgcc/1 -> SdbTxSigProc_Runnable_sys/107(AirSrcReqNgcc_AirSrcReqNgcc_read)
  AirTAftHvacDesFirstLe_AirTAftHvacDesFirstLe/1 -> SdbTxSigProc_Runnable_sys/108(AirTAftHvacDesFirstLe_AirTAftHvacDesFirstLe_read)
  AirTAftHvacDesFirstRi_AirTAftHvacDesFirstRi/1 -> SdbTxSigProc_Runnable_sys/109(AirTAftHvacDesFirstRi_AirTAftHvacDesFirstRi_read)
  AirTAftHvacDesRe_AirTAftHvacDesRe/1 -> SdbTxSigProc_Runnable_sys/110(AirTAftHvacDesRe_AirTAftHvacDesRe_read)
  AirTAftHvacDesSecLe_AirTAftHvacDesSecLe/1 -> SdbTxSigProc_Runnable_sys/111(AirTAftHvacDesSecLe_AirTAftHvacDesSecLe_read)
  AirTFromHeatgEstimdFirstLeForCCM_AirTFromHeatgEstimdFirstLeForCCM/1 -> SdbTxSigProc_Runnable_sys/112(AirTFromHeatgEstimdFirstLeForCCM_AirTFromHeatgEstimdFirstLeForCCM_read)
  AirTFromHeatgEstimdFirstRiForCCM_AirTFromHeatgEstimdFirstRiForCCM/1 -> SdbTxSigProc_Runnable_sys/113(AirTFromHeatgEstimdFirstRiForCCM_AirTFromHeatgEstimdFirstRiForCCM_read)
  AirTFromHeatgEstimdForCCM_AirTFromHeatgEstimd/1 -> SdbTxSigProc_Runnable_sys/114(AirTFromHeatgEstimdForCCM_AirTFromHeatgEstimd_read)
  AirTFromHeatgEstimdReForCCM_AirTFromHeatgEstimdReForCCM/1 -> SdbTxSigProc_Runnable_sys/115(AirTFromHeatgEstimdReForCCM_AirTFromHeatgEstimdReForCCM_read)
  AirTFromHeatgEstimdSecLeForCCM_AirTFromHeatgEstimdSecLeForCCM/1 -> SdbTxSigProc_Runnable_sys/116(AirTFromHeatgEstimdSecLeForCCM_AirTFromHeatgEstimdSecLeForCCM_read)
  AutOxygenSplyStsFrmClimaNgcc_AutOxygenSplyStsFrmClimaNgcc/1 -> SdbTxSigProc_Runnable_sys/117(AutOxygenSplyStsFrmClimaNgcc_AutOxygenSplyStsFrmClimaNgcc_read)
  CcmAACAirClngStsNgcc_CcmAACAirClngStsNgcc/1 -> SdbTxSigProc_Runnable_sys/118(CcmAACAirClngStsNgcc_CcmAACAirClngStsNgcc_read)
  CCMDataTraceZCUP1Ngcc_CCMDataTraceZCUP1Ngcc/1 -> SdbTxSigProc_Runnable_sys/119(CCMDataTraceZCUP1Ngcc_CCMDataTraceZCUP1Ngcc_read)
  CCMDataTraceZCUP2Ngcc_CCMDataTraceZCUP2Ngcc/1 -> SdbTxSigProc_Runnable_sys/120(CCMDataTraceZCUP2Ngcc_CCMDataTraceZCUP2Ngcc_read)
  CCMDataTraceZCUP3Ngcc_CCMDataTraceZCUP3Ngcc/1 -> SdbTxSigProc_Runnable_sys/121(CCMDataTraceZCUP3Ngcc_CCMDataTraceZCUP3Ngcc_read)
  CCMDataTraceZCUP4Ngcc_CCMDataTraceZCUP4Ngcc/1 -> SdbTxSigProc_Runnable_sys/122(CCMDataTraceZCUP4Ngcc_CCMDataTraceZCUP4Ngcc_read)
  CCMDataTraceZCUP5Ngcc_CCMDataTraceZCUP5Ngcc/1 -> SdbTxSigProc_Runnable_sys/123(CCMDataTraceZCUP5Ngcc_CCMDataTraceZCUP5Ngcc_read)
  CCMDataTraceZCUP6Ngcc_CCMDataTraceZCUP6Ngcc/1 -> SdbTxSigProc_Runnable_sys/124(CCMDataTraceZCUP6Ngcc_CCMDataTraceZCUP6Ngcc_read)
  CCMDataTraceZCUP7Ngcc_CCMDataTraceZCUP7Ngcc/1 -> SdbTxSigProc_Runnable_sys/125(CCMDataTraceZCUP7Ngcc_CCMDataTraceZCUP7Ngcc_read)
  CcmElecAirDirCrtlReqSecCnsLePosXNgcc_CcmElecAirDirCrtlReqSecCnsLePosXNgcc/1 -> SdbTxSigProc_Runnable_sys/126(CcmElecAirDirCrtlReqSecCnsLePosXNgcc_CcmElecAirDirCrtlReqSecCnsLePosXNgcc_read)
  CcmElecAirDirCrtlReqSecCnsLePosYNgcc_CcmElecAirDirCrtlReqSecCnsLePosYNgcc/1 -> SdbTxSigProc_Runnable_sys/127(CcmElecAirDirCrtlReqSecCnsLePosYNgcc_CcmElecAirDirCrtlReqSecCnsLePosYNgcc_read)
  CcmElecAirDirCrtlReqSecCnsRiPosXNgcc_CcmElecAirDirCrtlReqSecCnsRiPosXNgcc/1 -> SdbTxSigProc_Runnable_sys/128(CcmElecAirDirCrtlReqSecCnsRiPosXNgcc_CcmElecAirDirCrtlReqSecCnsRiPosXNgcc_read)
  CcmElecAirDirCrtlReqSecCnsRiPosYNgcc_CcmElecAirDirCrtlReqSecCnsRiPosYNgcc/1 -> SdbTxSigProc_Runnable_sys/129(CcmElecAirDirCrtlReqSecCnsRiPosYNgcc_CcmElecAirDirCrtlReqSecCnsRiPosYNgcc_read)
  CCMFragCh1OvrdDyNgcc_CCMFragCh1OvrdDyNgcc/1 -> SdbTxSigProc_Runnable_sys/130(CCMFragCh1OvrdDyNgcc_CCMFragCh1OvrdDyNgcc_read)
  CCMFragCh2OvrdDyNgcc_CCMFragCh2OvrdDyNgcc/1 -> SdbTxSigProc_Runnable_sys/131(CCMFragCh2OvrdDyNgcc_CCMFragCh2OvrdDyNgcc_read)
  CCMFragCh3OvrdDyNgcc_CCMFragCh3OvrdDyNgcc/1 -> SdbTxSigProc_Runnable_sys/132(CCMFragCh3OvrdDyNgcc_CCMFragCh3OvrdDyNgcc_read)
  CCMFragCh4OvrdDyNgcc_CCMFragCh4OvrdDyNgcc/1 -> SdbTxSigProc_Runnable_sys/133(CCMFragCh4OvrdDyNgcc_CCMFragCh4OvrdDyNgcc_read)
  CCMFragCh5OvrdDyNgcc_CCMFragCh5OvrdDyNgcc/1 -> SdbTxSigProc_Runnable_sys/134(CCMFragCh5OvrdDyNgcc_CCMFragCh5OvrdDyNgcc_read)
  CCMFragraCh1AvlTiPrecNgcc_CCMFragraCh1AvlTiPrecNgcc/1 -> SdbTxSigProc_Runnable_sys/135(CCMFragraCh1AvlTiPrecNgcc_CCMFragraCh1AvlTiPrecNgcc_read)
  CCMFragraCh1AvlTiPrecNgcc_CCMFragraCh1AvlTiPrecNgcc_IsUpdated/1 -> SdbTxSigProc_Runnable_sys/136(CCMFragraCh1AvlTiPrecNgcc_CCMFragraCh1AvlTiPrecNgcc_IsUpdated_read)
  CCMFragraCh2AvlTiPrecNgcc_CCMFragraCh2AvlTiPrecNgcc/1 -> SdbTxSigProc_Runnable_sys/137(CCMFragraCh2AvlTiPrecNgcc_CCMFragraCh2AvlTiPrecNgcc_read)
  CCMFragraCh2AvlTiPrecNgcc_CCMFragraCh2AvlTiPrecNgcc_IsUpdated/1 -> SdbTxSigProc_Runnable_sys/138(CCMFragraCh2AvlTiPrecNgcc_CCMFragraCh2AvlTiPrecNgcc_IsUpdated_read)
  CCMFragraCh3AvlTiPrecNgcc_CCMFragraCh3AvlTiPrecNgcc/1 -> SdbTxSigProc_Runnable_sys/139(CCMFragraCh3AvlTiPrecNgcc_CCMFragraCh3AvlTiPrecNgcc_read)
  CCMFragraCh3AvlTiPrecNgcc_CCMFragraCh3AvlTiPrecNgcc_IsUpdated/1 -> SdbTxSigProc_Runnable_sys/140(CCMFragraCh3AvlTiPrecNgcc_CCMFragraCh3AvlTiPrecNgcc_IsUpdated_read)
  CCMFragraCh4AvlTiPrecNgcc_CCMFragraCh4AvlTiPrecNgcc/1 -> SdbTxSigProc_Runnable_sys/141(CCMFragraCh4AvlTiPrecNgcc_CCMFragraCh4AvlTiPrecNgcc_read)
  CCMFragraCh4AvlTiPrecNgcc_CCMFragraCh4AvlTiPrecNgcc_IsUpdated/1 -> SdbTxSigProc_Runnable_sys/142(CCMFragraCh4AvlTiPrecNgcc_CCMFragraCh4AvlTiPrecNgcc_IsUpdated_read)
  CCMFragraCh5AvlTiPrecNgcc_CCMFragraCh5AvlTiPrecNgcc/1 -> SdbTxSigProc_Runnable_sys/143(CCMFragraCh5AvlTiPrecNgcc_CCMFragraCh5AvlTiPrecNgcc_read)
  CCMFragraCh5AvlTiPrecNgcc_CCMFragraCh5AvlTiPrecNgcc_IsUpdated/1 -> SdbTxSigProc_Runnable_sys/144(CCMFragraCh5AvlTiPrecNgcc_CCMFragraCh5AvlTiPrecNgcc_IsUpdated_read)
  CcmWbmMasterReqLeLin1Ngcc_CcmWbmMasterReqLeLin1Ngcc/1 -> SdbTxSigProc_Runnable_sys/145(CcmWbmMasterReqLeLin1Ngcc_CcmWbmMasterReqLeLin1Ngcc_read)
  CcmWbmMasterReqRiLin1Ngcc_CcmWbmMasterReqRiLin1Ngcc/1 -> SdbTxSigProc_Runnable_sys/146(CcmWbmMasterReqRiLin1Ngcc_CcmWbmMasterReqRiLin1Ngcc_read)
  CdsnProblty_CdsnProblty/1 -> SdbTxSigProc_Runnable_sys/147(CdsnProblty_CdsnProblty_read)
  ChgFilElmRstNgcc_ChgFilElmRstNgcc/1 -> SdbTxSigProc_Runnable_sys/148(ChgFilElmRstNgcc_ChgFilElmRstNgcc_read)
  ClimaAtRowSecActvNgcc_ClimaAtRowSecActv/1 -> SdbTxSigProc_Runnable_sys/149(ClimaAtRowSecActvNgcc_ClimaAtRowSecActv_read)
  ClimaAtRowSecActvNgcc_ClimaAtRowSecActvLe/1 -> SdbTxSigProc_Runnable_sys/150(ClimaAtRowSecActvNgcc_ClimaAtRowSecActvLe_read)
  ClimaAtRowSecActvNgcc_ClimaAtRowSecActvRi/1 -> SdbTxSigProc_Runnable_sys/151(ClimaAtRowSecActvNgcc_ClimaAtRowSecActvRi_read)
  ClimaCtrlActvNgcc_ClimaCtrlActv/1 -> SdbTxSigProc_Runnable_sys/152(ClimaCtrlActvNgcc_ClimaCtrlActv_read)
  ClimaManModCmftPopUpReqNgcc_ClimaManModCmftPopUpReqNgcc/1 -> SdbTxSigProc_Runnable_sys/153(ClimaManModCmftPopUpReqNgcc_ClimaManModCmftPopUpReqNgcc_read)
  CmptmtAirDistbnRspNgcc_CmptmtAirDistbnRspNgcc/1 -> SdbTxSigProc_Runnable_sys/154(CmptmtAirDistbnRspNgcc_CmptmtAirDistbnRspNgcc_read)
  CmptmtAirDistbnRspNgcc_CmptmtAirDistbnRspNgcc_IsUpdated/1 -> SdbTxSigProc_Runnable_sys/155(CmptmtAirDistbnRspNgcc_CmptmtAirDistbnRspNgcc_IsUpdated_read)
  CmptmtOxygenConcLvlFrmClimaNgcc_CmptmtOxygenConcLvlFrmClimaNgcc/1 -> SdbTxSigProc_Runnable_sys/156(CmptmtOxygenConcLvlFrmClimaNgcc_CmptmtOxygenConcLvlFrmClimaNgcc_read)
  CmptmtOxygenConcVluFrmClimaNgcc_CmptmtOxygenConcVluFrmClimaNgcc/1 -> SdbTxSigProc_Runnable_sys/157(CmptmtOxygenConcVluFrmClimaNgcc_CmptmtOxygenConcVluFrmClimaNgcc_read)
  CoupldCtrlErrAtRowFirstLeNgcc_CoupldCtrlErrAtRowFirstLe/1 -> SdbTxSigProc_Runnable_sys/158(CoupldCtrlErrAtRowFirstLeNgcc_CoupldCtrlErrAtRowFirstLe_read)
  CoupldCtrlErrAtRowFirstRiNgcc_CoupldCtrlErrAtRowFirstRi/1 -> SdbTxSigProc_Runnable_sys/159(CoupldCtrlErrAtRowFirstRiNgcc_CoupldCtrlErrAtRowFirstRi_read)
  CoupldCtrlErrAtRowSecLeNgcc_CoupldCtrlErrAtRowSecLe/1 -> SdbTxSigProc_Runnable_sys/160(CoupldCtrlErrAtRowSecLeNgcc_CoupldCtrlErrAtRowSecLe_read)
  CoupldCtrlErrAtRowSecRiNgcc_CoupldCtrlErrAtRowSecRi/1 -> SdbTxSigProc_Runnable_sys/161(CoupldCtrlErrAtRowSecRiNgcc_CoupldCtrlErrAtRowSecRi_read)
  DutyCmdForMosBlw_DutyCmdForMosBlw/1 -> SdbTxSigProc_Runnable_sys/162(DutyCmdForMosBlw_DutyCmdForMosBlw_read)
  DutyCmdForPWMBlw_DutyCmdForPWMBlw/1 -> SdbTxSigProc_Runnable_sys/163(DutyCmdForPWMBlw_DutyCmdForPWMBlw_read)
  ExtVentToastFrmClima_Ngcc_ExtVentToastFrmClima/1 -> SdbTxSigProc_Runnable_sys/164(ExtVentToastFrmClima_Ngcc_ExtVentToastFrmClima_read)
  FrntClimaManModCmftStsNgcc_FrntClimaManModCmftStsNgcc/1 -> SdbTxSigProc_Runnable_sys/165(FrntClimaManModCmftStsNgcc_FrntClimaManModCmftStsNgcc_read)
  HighTempCleanRunningStsNgcc_HighTempCleanRunningStsNgcc/1 -> SdbTxSigProc_Runnable_sys/166(HighTempCleanRunningStsNgcc_HighTempCleanRunningStsNgcc_read)
  HighTempCleanSettingStsNgcc_HighTempCleanSettingStsNgcc/1 -> SdbTxSigProc_Runnable_sys/167(HighTempCleanSettingStsNgcc_HighTempCleanSettingStsNgcc_read)